simulink model "TosAeb"  (R2021a)
settings: Solver=FixedStepDiscrete  StartTime=0.0  StopTime=inf  FixedStep=0.01  RelTol=1e-3
undefined symbols (not defined in the file or in its folder): ACCModSts, AccSts1, AEB_ObjectMovingOrientation, AEB_ObjectMovingState, CN_BTCActive, CN_CurrentGearR, CN_CurrentGearR_DHT, CN_PTCActive, CN_VDCActive, CN_VSC_EspOff, CN_VSC_FCW_AEB_Error, CN_VSC_FCW_AEB_NoDispay, CN_VSC_FCW_AEB_PerfmDgdtn, CN_VSC_FCW_AEB_TempUnAvlbl, CN_VSC_VehStandstill, CNaccelAvoidMax, CNaccelAvoidMin, CNbrakePedalPress, CNcomfortStrLeftAccelMax, CNcomfortStrLeftAccelMin, CNcomfortStrRightAccelMax, CNcomfortStrRightAccelMin, CNDrvDoorOpen, CNDrvModeEco, CNDrvModeNorm, CNDrvModeOther, CNDrvModeSport, CNDrvSBRreminder, CNNull, CNobjInvalidID, CNPassDoorOpen, CNPassSBRreminder, CNPEBSAccelNegInf, CNPEBSAccelNull, CNPEBSTimeInf, CNPEBSTimeNull, CNSTOPMAX, CNSTOPNULL, CNsubjWidth, CNSwtOFF, CNSwtON, CNTTCMAX, CNTTCNULL, CNVehConfigAEBCycNone, CNVehConfigAEBNone, CNVehConfigAEBPedNone, CNVehConfigFCWNone, CNVehConfigJANone, CollisionSeverity, FALSE, FCWSnvty, JACycObjCalcInfoDefaultParams, JAPedObjCalcInfoDefaultParams, JAVehObjCalcInfoDefaultParams, latCycObjCalcInfoDefaultParams, latPedObjCalcInfoDefaultParams, longObjCalcInfoDefaultParams, ModSts, movingStatus, ObjectType ... (+1112)
libraries: simulink x3031, AEBSFunctionLib x50, Ta_Library x33, AEBSStateMachineLib x23, Mylibrary x12, simulink_extras x7, ObjSelect_lib x3, dspstat3 x2
(part 7 of 102)

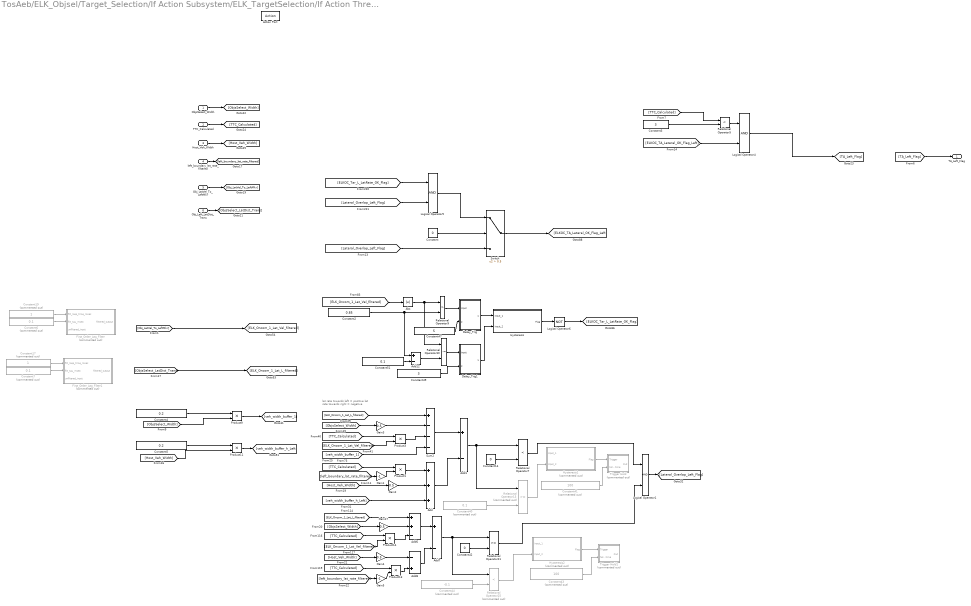
== system TosAeb/ELK_Objsel/Target_Selection/If Action Subsystem/ELK_TargetSelection/If Action Threat_Assessment_Left1 ==
  note: lat rate towards left = positive
      | lat rate towards right = negative
  ObjsSelect_Width : Inport
  TTC_Calculated : Inport  Port=2
  Host_Veh_Width : Inport  Port=3
  left_boundary_lat_rate_filtered : Inport  Port=4
  Obj_LatVel_To_LeftMkr : Inport  Port=5
  Obj_Left_LatDist_Trans : Inport  Port=6
  Action Port : ActionPort  ActionPortLabel=if(u1 ~= 0)
  Abs : Abs  Description=_GWMH9P4.ELK.000_40_
  Add : Sum  Description=_GWMH9P5.ELK.000_14_  Inputs=+++
  Add1 : Sum  Description=_GWMH9P5.ELK.000_15_  Inputs=+-
  Add12 : Sum  Description=_GWMH9P5.ELK.000_18_  Inputs=+-
  Add6 : Sum  Description=_GWMH9P5.ELK.000_22_  Inputs=++-
  Add7 : Sum  Description=_GWMH9P5.ELK.000_23_  Inputs=+-
  Add8 : Sum  Description=_GWMH9P5.ELK.000_24_  Inputs=-+
  Constant : Constant  Value=0
  Constant14 : Constant  Description=_GWMH9P4.ELK.000_42_  Value=0
  Constant17 : Constant  Description=_GWMH9P5.ELK.000_26_  Commented=on  OutDataTypeStr=single
  Constant19 : Constant  Description=_GWMH9P5.ELK.000_27_  Commented=on  OutDataTypeStr=single
  Constant2 : Constant  Description=_GWMH9P5.ELK.000_52_  Value=0.85
  Constant3 : Constant  Description=_GWMH9P5.ELK.000_38_  Value=3
  Constant4 : Constant  Description=_GWMH9P5.ELK.000_45_  Value=0.2
  Constant40 : Constant  Description=_GWMH9P5.ELK.000_41_  Commented=on  Value=0.1  mask(TL_Constant){data={'date',738384.81607121532}}
      settings: CopyFcn=if exist('tl_reset_reviewflag','file')==2,tl_reset_reviewflag(gcbh);end  DeleteFcn=if exist('delete_tlblock','file')==2,delete_tlblock(gcbh);end  NameChangeFcn=if exist('rename_tlblock','file')==2,rename_tlblock(gcbh);end  OpenFcn=if exist('tl_open_blockdlg','file')==2,tl_open_blockdlg(gcbh);else,open_system(gcbh,'parameter');end
      mask Help: eval(['if exist(''tl_online_help'',''file'') == 2, tl_online_help(''Topic'',''TL_Constant''),else, web([''jar:file:///'',matlabroot,''/help/toolbox/simulink/help.jar!/slref/' lower(get_param(gcbh,'Blocktype')) '.html''], ''-helpbrowser''),end'])
      mask Display: plot([-0.1, 1.05, NaN, -0.1, 1.05],[.01, .01, NaN, .99, .99]);
  Constant41 : Constant  Description=_GWMH9P5.ELK.000_42_  Commented=on  Value=100  mask(TL_Constant){data={'date',738384.81607467588}}
      settings: CopyFcn=if exist('tl_reset_reviewflag','file')==2,tl_reset_reviewflag(gcbh);end  DeleteFcn=if exist('delete_tlblock','file')==2,delete_tlblock(gcbh);end  NameChangeFcn=if exist('rename_tlblock','file')==2,rename_tlblock(gcbh);end  OpenFcn=if exist('tl_open_blockdlg','file')==2,tl_open_blockdlg(gcbh);else,open_system(gcbh,'parameter');end
      mask Help: eval(['if exist(''tl_online_help'',''file'') == 2, tl_online_help(''Topic'',''TL_Constant''),else, web([''jar:file:///'',matlabroot,''/help/toolbox/simulink/help.jar!/slref/' lower(get_param(gcbh,'Blocktype')) '.html''], ''-helpbrowser''),end'])
      mask Display: plot([-0.1, 1.05, NaN, -0.1, 1.05],[.01, .01, NaN, .99, .99]);
  Constant42 : Constant  Description=_GWMH9P5.ELK.000_43_  Value=0
  Constant43 : Constant  Description=_GWMH9P5.ELK.000_44_  Commented=on  Value=100  mask(TL_Constant){data={'date',738384.81608211808}}
      settings: CopyFcn=if exist('tl_reset_reviewflag','file')==2,tl_reset_reviewflag(gcbh);end  DeleteFcn=if exist('delete_tlblock','file')==2,delete_tlblock(gcbh);end  NameChangeFcn=if exist('rename_tlblock','file')==2,rename_tlblock(gcbh);end  OpenFcn=if exist('tl_open_blockdlg','file')==2,tl_open_blockdlg(gcbh);else,open_system(gcbh,'parameter');end
      mask Help: eval(['if exist(''tl_online_help'',''file'') == 2, tl_online_help(''Topic'',''TL_Constant''),else, web([''jar:file:///'',matlabroot,''/help/toolbox/simulink/help.jar!/slref/' lower(get_param(gcbh,'Blocktype')) '.html''], ''-helpbrowser''),end'])
      mask Display: plot([-0.1, 1.05, NaN, -0.1, 1.05],[.01, .01, NaN, .99, .99]);
  Constant44 : Constant  Description=_GWMH9P5.ELK.000_45_  Commented=on  Value=-0.1  mask(TL_Constant){data={'date',738384.81608572917}}
      settings: CopyFcn=if exist('tl_reset_reviewflag','file')==2,tl_reset_reviewflag(gcbh);end  DeleteFcn=if exist('delete_tlblock','file')==2,delete_tlblock(gcbh);end  NameChangeFcn=if exist('rename_tlblock','file')==2,rename_tlblock(gcbh);end  OpenFcn=if exist('tl_open_blockdlg','file')==2,tl_open_blockdlg(gcbh);else,open_system(gcbh,'parameter');end
      mask Help: eval(['if exist(''tl_online_help'',''file'') == 2, tl_online_help(''Topic'',''TL_Constant''),else, web([''jar:file:///'',matlabroot,''/help/toolbox/simulink/help.jar!/slref/' lower(get_param(gcbh,'Blocktype')) '.html''], ''-helpbrowser''),end'])
      mask Display: plot([-0.1, 1.05, NaN, -0.1, 1.05],[.01, .01, NaN, .99, .99]);
  Constant47 : Constant  Description=_GWMH9P5.ELK.000_48_  Value=5
  Constant49 : Constant  Description=_GWMH9P5.ELK.000_50_  Value=3
  Constant5 : Constant  Description=_GWMH9P5.ELK.000_45_  Value=0.2
  Constant51 : Constant  Description=_GWMH9P5.ELK.000_52_  Value=0.1
  Constant6 : Constant  Description=_GWMH9P5.ELK.000_27_  Commented=on  Value=0.1  OutDataTypeStr=single
  Constant7 : Constant  Description=_GWMH9P5.ELK.000_27_  Commented=on  Value=0.1  OutDataTypeStr=single
  Delay_Trig : Subsystem
  Delay_Trig1 : Subsystem
  First_Order_Lag_Filter : Subsystem  Commented=on
  First_Order_Lag_Filter1 : Subsystem  Commented=on
  Gain1 : Gain  Description=_GWMH9P4.ELK.000_64_  Gain=-1
  Gain17 : Gain  Description=_GWMH9P5.ELK.000_80_  Gain=0.5
  Gain2 : Gain  Description=_GWMH9P4.ELK.000_64_  Gain=0.5
  Gain3 : Gain  Description=_GWMH9P4.ELK.000_64_  Gain=0.5
  Gain4 : Gain  Description=_GWMH9P4.ELK.000_64_  Gain=0.5
  Gain5 : Gain  Description=_GWMH9P4.ELK.000_64_  Gain=-1
  Hysteresis : Stateflow chart  TreatAsAtomicUnit=on
  Hysteresis1 : Stateflow chart  Commented=on  TreatAsAtomicUnit=on
  Hysteresis2 : Stateflow chart  Commented=on  TreatAsAtomicUnit=on
  Logical Operator1 : Logic  Description=_GWMH9P5.ELK.000_86_  AllPortsSameDT=off  OutDataTypeStr=boolean
  Logical Operator4 : Logic  Description=_GWMH9P4.ELK.000_68_  AllPortsSameDT=off  OutDataTypeStr=boolean
  Logical Operator5 : Logic  Description=_GWMH9P4.ELK.000_68_  AllPortsSameDT=off  OutDataTypeStr=boolean
  Logical Operator6 : Logic  Description=_GWMH9P5.ELK.000_88_  Operator=NOT  Inputs=1  AllPortsSameDT=off  OutDataTypeStr=boolean
  Product1 : Product  Description=_GWMH9P4.ELK.000_71_  RndMeth=Zero
  Product11 : Product  Description=_GWMH9P5.ELK.000_91_
  Product13 : Product  Description=_GWMH9P5.ELK.000_93_  RndMeth=Zero
  Product14 : Product  Description=_GWMH9P5.ELK.000_94_  RndMeth=Zero
  Product3 : Product  Description=_GWMH9P4.ELK.000_73_  RndMeth=Zero
  Product9 : Product  Description=_GWMH9P5.ELK.000_102_
  Relational Operator13 : RelationalOperator  Description=_GWMH9P5.ELK.000_106_  Commented=on  OutDataTypeStr=boolean  RndMeth=Nearest  mask(TL_RelationalOperator){data={'date',738384.81682939816}}
      settings: DeleteFcn=if exist('delete_tlblock','file')==2,delete_tlblock(gcbh);end  NameChangeFcn=if exist('rename_tlblock','file')==2,rename_tlblock(gcbh);end  OpenFcn=if exist('tl_open_blockdlg','file')==2,tl_open_blockdlg(gcbh);else,open_system(gcbh,'parameter');end
      mask Help: eval(['if exist(''tl_online_help'',''file'') == 2, tl_online_help(''Topic'',''TL_RelationalOperator''),else, web([''jar:file:///'',matlabroot,''/help/toolbox/simulink/help.jar!/slref/' lower(get_param(gcbh,'Blocktype')) '.html''], ''-helpbrowser''),end'])
      mask Display: plot([-0.1, 1.05, NaN, -0.1, 1.05],[.01, .01, NaN, .99, .99]);
  Relational Operator21 : RelationalOperator  Description=_GWMH9P5.ELK.000_108_  OutDataTypeStr=boolean  RndMeth=Nearest
  Relational Operator25 : RelationalOperator  Description=_GWMH9P5.ELK.000_109_  Commented=on  Operator=<  OutDataTypeStr=boolean  RndMeth=Nearest  mask(TL_RelationalOperator){data={'date',738384.8168361343}}
      settings: DeleteFcn=if exist('delete_tlblock','file')==2,delete_tlblock(gcbh);end  NameChangeFcn=if exist('rename_tlblock','file')==2,rename_tlblock(gcbh);end  OpenFcn=if exist('tl_open_blockdlg','file')==2,tl_open_blockdlg(gcbh);else,open_system(gcbh,'parameter');end
      mask Help: eval(['if exist(''tl_online_help'',''file'') == 2, tl_online_help(''Topic'',''TL_RelationalOperator''),else, web([''jar:file:///'',matlabroot,''/help/toolbox/simulink/help.jar!/slref/' lower(get_param(gcbh,'Blocktype')) '.html''], ''-helpbrowser''),end'])
      mask Display: plot([-0.1, 1.05, NaN, -0.1, 1.05],[.01, .01, NaN, .99, .99]);
  Relational Operator30 : RelationalOperator  Description=_GWMH9P5.ELK.000_112_  Operator=<=  OutDataTypeStr=boolean  RndMeth=Nearest
  Relational Operator5 : RelationalOperator  Description=_GWMH9P3.ELK.000_91_  Operator=<  OutDataTypeStr=boolean  RndMeth=Nearest
  Relational Operator7 : RelationalOperator  Description=_GWMH9P4.ELK.000_81_  Operator=<  OutDataTypeStr=boolean  RndMeth=Nearest
  Relational Operator9 : RelationalOperator  Description=_GWMH9P5.ELK.000_114_  Operator=>  OutDataTypeStr=boolean  RndMeth=Nearest
  Sum2 : Sum  Description=_GWMH9P4.ELK.000_83_  Inputs=+---
  Switch : Switch  Criteria=u2 > Threshold  Threshold=0.5
  Trigger Hold : Subsystem  Commented=on
  Trigger Hold1 : Subsystem  Commented=on
  TA_Left_Flag : Outport
  wires:
  Logical Operator6/1 -> [ELKOC_Tar_L_LatRate_OK_Flag]
  [Lateral_Overlap_Left_Flag] -> Switch/3
  Constant/1 -> Switch/2
  Switch/1 -> [ELKOC_TA_Lateral_OK_Flag_Left]
  Logical Operator5/1 -> Switch/1
  Gain5/1 -> Product13/2
  [Host_Veh_Width] -> Gain4/1
  Gain2/1 -> Add/2
  Gain1/1 -> Product1/2
  Obj_Left_LatDist_Trans/1 -> [ObjsSelect_LatDist_Trans]
  Delay_Trig/1(Output) -> Hysteresis/1(Input_1)
  Constant5/1 -> Product11/1
  [Host_Veh_Width] -> Product11/2
  Product11/1 -> [veh_width_buffer_h_Left]
  Product9/1 -> [veh_width_buffer_1]
  Constant4/1 -> Product9/1
  [ObjsSelect_Width] -> Product9/2
  [ObjsSelect_LatDist_Trans] -> [ELK_Oncom_1_Lat_L_filtered]
  [TTC_Calculated] -> Product1/1
  [ObjsSelect_Width] -> Gain3/1
  [left_boundary_lat_rate_filtered] -> Gain1/1
  Constant14/1 -> Relational Operator7/2
  [TTC_Calculated] -> Product3/1
  [ELK_Oncom_1_Lat_Vel_filtered] -> Product3/2
  [ELK_Oncom_1_Lat_L_filtered] -> Sum2/1
  Gain3/1 -> Sum2/2
  Product3/1 -> Sum2/3
  [veh_width_buffer_1] -> Sum2/4
  Product1/1 -> Add/1
  [Host_Veh_Width] -> Gain2/1
  [veh_width_buffer_h_Left] -> Add/3
  Sum2/1 -> Add1/1
  Add/1 -> Add1/2
  Add1/1 -> Relational Operator13/1, Relational Operator7/1
  Logical Operator1/1 -> [Lateral_Overlap_Left_Flag]
  Hysteresis1/1(Flag) -> Trigger Hold/1(Trigger)
  Relational Operator7/1 -> Logical Operator1/1
  Constant40/1 -> Relational Operator13/2
  Relational Operator13/1 -> Hysteresis1/2(Input_2)
  Constant41/1 -> Trigger Hold/2(hold_time)
  [ObjsSelect_Width] -> Gain17/1
  Gain17/1 -> Add6/2
  [TTC_Calculated] -> Product14/1
  [ELK_Oncom_1_Lat_Vel_filtered] -> Product14/2
  Product14/1 -> Add6/3
  Add6/1 -> Add7/1
  [TTC_Calculated] -> Product13/1
  [left_boundary_lat_rate_filtered] -> Gain5/1
  Add7/1 -> Relational Operator25/1, Relational Operator21/1
  Constant42/1 -> Relational Operator21/2
  [ELK_Oncom_1_Lat_L_filtered] -> Add6/1
  Product13/1 -> Add8/2
  Gain4/1 -> Add8/1
  Add8/1 -> Add7/2
  Constant44/1 -> Relational Operator25/2
  Relational Operator21/1 -> Logical Operator1/2
  Relational Operator25/1 -> Hysteresis2/2(Input_2)
  Hysteresis2/1(Flag) -> Trigger Hold1/1(Trigger)
  Constant43/1 -> Trigger Hold1/2(hold_time)
  [Obj_LatVel_To_LeftMkr] -> [ELK_Oncom_1_Lat_Vel_filtered]
  [ELK_Oncom_1_Lat_Vel_filtered] -> Abs/1
  Constant2/1 -> Add12/1, Relational Operator9/2
  Constant47/1 -> Delay_Trig/2(Delay_Cnt)
  Relational Operator30/1 -> Delay_Trig1/1(Input)
  Delay_Trig1/1(Output) -> Hysteresis/2(Input_2)
  Constant49/1 -> Delay_Trig1/2(Delay_Cnt)
  Abs/1 -> Relational Operator30/1, Relational Operator9/1
  Add12/1 -> Relational Operator30/2
  Constant51/1 -> Add12/2
  Relational Operator9/1 -> Delay_Trig/1(Input)
  Hysteresis/1(Flag) -> Logical Operator6/1
  Obj_LatVel_To_LeftMkr/1 -> [Obj_LatVel_To_LeftMkr]
  left_boundary_lat_rate_filtered/1 -> [left_boundary_lat_rate_filtered]
  Host_Veh_Width/1 -> [Host_Veh_Width]
  TTC_Calculated/1 -> [TTC_Calculated]
  ObjsSelect_Width/1 -> [ObjsSelect_Width]
  [TA_Left_Flag] -> TA_Left_Flag/1
  [Lateral_Overlap_Left_Flag] -> Logical Operator5/2
  [ELKOC_Tar_L_LatRate_OK_Flag] -> Logical Operator5/1
  Relational Operator5/1 -> Logical Operator4/1
  [TTC_Calculated] -> Relational Operator5/1
  [ELKOC_TA_Lateral_OK_Flag_Left] -> Logical Operator4/2
  Constant3/1 -> Relational Operator5/2
  Logical Operator4/1 -> [TA_Left_Flag]
  Constant6/1 -> First_Order_Lag_Filter/2(filt_tau_msec)
  Constant19/1 -> First_Order_Lag_Filter/1(filt_loop_time_msec)
  Constant7/1 -> First_Order_Lag_Filter1/2(filt_tau_msec)
  Constant17/1 -> First_Order_Lag_Filter1/1(filt_loop_time_msec)

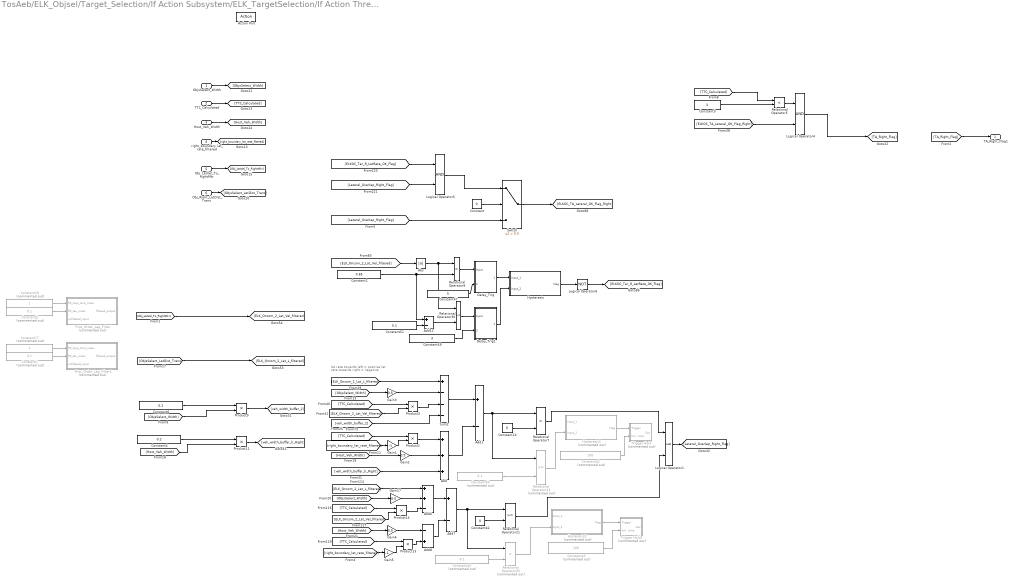
== system TosAeb/ELK_Objsel/Target_Selection/If Action Subsystem/ELK_TargetSelection/If Action Threat_Assessment_Right ==
  note: lat rate towards left = positive
      | lat rate towards right = negative
  ObjsSelect_Width : Inport
  TTC_Calculated : Inport  Port=2
  Host_Veh_Width : Inport  Port=3
  right_boundary_lat_rate_filtered : Inport  Port=4
  Obj_LatVel_To_RightMkr : Inport  Port=5
  Obj_Right_LatDist_Trans : Inport  Port=6
  Action Port : ActionPort  ActionPortLabel=if(u1 ~= 0)
  Abs : Abs  Description=_GWMH9P4.ELK.000_40_
  Add : Sum  Description=_GWMH9P5.ELK.000_14_  Inputs=+++
  Add1 : Sum  Description=_GWMH9P5.ELK.000_15_  Inputs=+-
  Add12 : Sum  Description=_GWMH9P5.ELK.000_18_  Inputs=+-
  Add6 : Sum  Description=_GWMH9P5.ELK.000_22_  Inputs=++-
  Add7 : Sum  Description=_GWMH9P5.ELK.000_23_  Inputs=+-
  Add8 : Sum  Description=_GWMH9P5.ELK.000_24_  Inputs=-+
  Constant : Constant  Value=0
  Constant14 : Constant  Description=_GWMH9P4.ELK.000_42_  Value=0
  Constant17 : Constant  Description=_GWMH9P5.ELK.000_26_  Commented=on
  Constant19 : Constant  Description=_GWMH9P5.ELK.000_27_  Commented=on
  Constant2 : Constant  Description=_GWMH9P5.ELK.000_52_  Value=0.85
  Constant3 : Constant  Description=_GWMH9P5.ELK.000_38_  Value=3
  Constant4 : Constant  Description=_GWMH9P5.ELK.000_52_  Value=0.2
  Constant40 : Constant  Description=_GWMH9P5.ELK.000_41_  Commented=on  Value=0.1  mask(TL_Constant){data={'date',738384.85978439811}}
      settings: CopyFcn=if exist('tl_reset_reviewflag','file')==2,tl_reset_reviewflag(gcbh);end  DeleteFcn=if exist('delete_tlblock','file')==2,delete_tlblock(gcbh);end  NameChangeFcn=if exist('rename_tlblock','file')==2,rename_tlblock(gcbh);end  OpenFcn=if exist('tl_open_blockdlg','file')==2,tl_open_blockdlg(gcbh);else,open_system(gcbh,'parameter');end
      mask Help: eval(['if exist(''tl_online_help'',''file'') == 2, tl_online_help(''Topic'',''TL_Constant''),else, web([''jar:file:///'',matlabroot,''/help/toolbox/simulink/help.jar!/slref/' lower(get_param(gcbh,'Blocktype')) '.html''], ''-helpbrowser''),end'])
      mask Display: plot([-0.1, 1.05, NaN, -0.1, 1.05],[.01, .01, NaN, .99, .99]);
  Constant41 : Constant  Description=_GWMH9P5.ELK.000_42_  Commented=on  Value=100  mask(TL_Constant){data={'date',738384.85978776624}}
      settings: CopyFcn=if exist('tl_reset_reviewflag','file')==2,tl_reset_reviewflag(gcbh);end  DeleteFcn=if exist('delete_tlblock','file')==2,delete_tlblock(gcbh);end  NameChangeFcn=if exist('rename_tlblock','file')==2,rename_tlblock(gcbh);end  OpenFcn=if exist('tl_open_blockdlg','file')==2,tl_open_blockdlg(gcbh);else,open_system(gcbh,'parameter');end
      mask Help: eval(['if exist(''tl_online_help'',''file'') == 2, tl_online_help(''Topic'',''TL_Constant''),else, web([''jar:file:///'',matlabroot,''/help/toolbox/simulink/help.jar!/slref/' lower(get_param(gcbh,'Blocktype')) '.html''], ''-helpbrowser''),end'])
      mask Display: plot([-0.1, 1.05, NaN, -0.1, 1.05],[.01, .01, NaN, .99, .99]);
  Constant42 : Constant  Description=_GWMH9P5.ELK.000_43_  Value=0
  Constant43 : Constant  Description=_GWMH9P5.ELK.000_44_  Commented=on  Value=100  mask(TL_Constant){data={'date',738384.85979500005}}
      settings: CopyFcn=if exist('tl_reset_reviewflag','file')==2,tl_reset_reviewflag(gcbh);end  DeleteFcn=if exist('delete_tlblock','file')==2,delete_tlblock(gcbh);end  NameChangeFcn=if exist('rename_tlblock','file')==2,rename_tlblock(gcbh);end  OpenFcn=if exist('tl_open_blockdlg','file')==2,tl_open_blockdlg(gcbh);else,open_system(gcbh,'parameter');end
      mask Help: eval(['if exist(''tl_online_help'',''file'') == 2, tl_online_help(''Topic'',''TL_Constant''),else, web([''jar:file:///'',matlabroot,''/help/toolbox/simulink/help.jar!/slref/' lower(get_param(gcbh,'Blocktype')) '.html''], ''-helpbrowser''),end'])
      mask Display: plot([-0.1, 1.05, NaN, -0.1, 1.05],[.01, .01, NaN, .99, .99]);
  Constant44 : Constant  Description=_GWMH9P5.ELK.000_45_  Commented=on  Value=-0.1  mask(TL_Constant){data={'date',738384.85979850695}}
      settings: CopyFcn=if exist('tl_reset_reviewflag','file')==2,tl_reset_reviewflag(gcbh);end  DeleteFcn=if exist('delete_tlblock','file')==2,delete_tlblock(gcbh);end  NameChangeFcn=if exist('rename_tlblock','file')==2,rename_tlblock(gcbh);end  OpenFcn=if exist('tl_open_blockdlg','file')==2,tl_open_blockdlg(gcbh);else,open_system(gcbh,'parameter');end
      mask Help: eval(['if exist(''tl_online_help'',''file'') == 2, tl_online_help(''Topic'',''TL_Constant''),else, web([''jar:file:///'',matlabroot,''/help/toolbox/simulink/help.jar!/slref/' lower(get_param(gcbh,'Blocktype')) '.html''], ''-helpbrowser''),end'])
      mask Display: plot([-0.1, 1.05, NaN, -0.1, 1.05],[.01, .01, NaN, .99, .99]);
  Constant47 : Constant  Description=_GWMH9P5.ELK.000_48_  Value=5
  Constant49 : Constant  Description=_GWMH9P5.ELK.000_50_  Value=3
  Constant5 : Constant  Description=_GWMH9P5.ELK.000_27_  Commented=on  Value=0.1
  Constant51 : Constant  Description=_GWMH9P5.ELK.000_52_  Value=0.1
  Constant6 : Constant  Description=_GWMH9P5.ELK.000_52_  Value=0.2
  Constant7 : Constant  Description=_GWMH9P5.ELK.000_27_  Commented=on  Value=0.1
  Delay_Trig : Subsystem
  Delay_Trig1 : Subsystem
  First_Order_Lag_Filter : Subsystem  Commented=on
  First_Order_Lag_Filter1 : Subsystem  Commented=on
  Gain1 : Gain  Description=_GWMH9P4.ELK.000_64_  Gain=-1
  Gain17 : Gain  Description=_GWMH9P5.ELK.000_80_  Gain=0.5
  Gain2 : Gain  Description=_GWMH9P4.ELK.000_64_  Gain=0.5
  Gain3 : Gain  Description=_GWMH9P4.ELK.000_64_  Gain=0.5
  Gain4 : Gain  Description=_GWMH9P4.ELK.000_64_  Gain=0.5
  Gain5 : Gain  Description=_GWMH9P4.ELK.000_64_  Gain=-1
  Hysteresis : Stateflow chart  TreatAsAtomicUnit=on
  Hysteresis1 : Stateflow chart  Commented=on  TreatAsAtomicUnit=on
  Hysteresis2 : Stateflow chart  Commented=on  TreatAsAtomicUnit=on
  Logical Operator1 : Logic  Description=_GWMH9P5.ELK.000_86_  AllPortsSameDT=off  OutDataTypeStr=boolean
  Logical Operator4 : Logic  Description=_GWMH9P4.ELK.000_68_  AllPortsSameDT=off  OutDataTypeStr=boolean
  Logical Operator5 : Logic  Description=_GWMH9P4.ELK.000_68_  AllPortsSameDT=off  OutDataTypeStr=boolean
  Logical Operator6 : Logic  Description=_GWMH9P5.ELK.000_88_  Operator=NOT  Inputs=1  AllPortsSameDT=off  OutDataTypeStr=boolean
  Product1 : Product  Description=_GWMH9P4.ELK.000_71_  RndMeth=Zero
  Product11 : Product  Description=_GWMH9P5.ELK.000_91_
  Product13 : Product  Description=_GWMH9P5.ELK.000_93_  RndMeth=Zero
  Product14 : Product  Description=_GWMH9P5.ELK.000_94_  RndMeth=Zero
  Product3 : Product  Description=_GWMH9P4.ELK.000_73_  RndMeth=Zero
  Product9 : Product  Description=_GWMH9P5.ELK.000_102_
  Relational Operator13 : RelationalOperator  Description=_GWMH9P5.ELK.000_106_  Commented=on  OutDataTypeStr=boolean  RndMeth=Nearest  mask(TL_RelationalOperator){data={'date',738384.85993935179}}
      settings: DeleteFcn=if exist('delete_tlblock','file')==2,delete_tlblock(gcbh);end  NameChangeFcn=if exist('rename_tlblock','file')==2,rename_tlblock(gcbh);end  OpenFcn=if exist('tl_open_blockdlg','file')==2,tl_open_blockdlg(gcbh);else,open_system(gcbh,'parameter');end
      mask Help: eval(['if exist(''tl_online_help'',''file'') == 2, tl_online_help(''Topic'',''TL_RelationalOperator''),else, web([''jar:file:///'',matlabroot,''/help/toolbox/simulink/help.jar!/slref/' lower(get_param(gcbh,'Blocktype')) '.html''], ''-helpbrowser''),end'])
      mask Display: plot([-0.1, 1.05, NaN, -0.1, 1.05],[.01, .01, NaN, .99, .99]);
  Relational Operator21 : RelationalOperator  Description=_GWMH9P5.ELK.000_108_  OutDataTypeStr=boolean  RndMeth=Nearest
  Relational Operator25 : RelationalOperator  Description=_GWMH9P5.ELK.000_109_  Commented=on  Operator=<  OutDataTypeStr=boolean  RndMeth=Nearest  mask(TL_RelationalOperator){data={'date',738384.85994590283}}
      settings: DeleteFcn=if exist('delete_tlblock','file')==2,delete_tlblock(gcbh);end  NameChangeFcn=if exist('rename_tlblock','file')==2,rename_tlblock(gcbh);end  OpenFcn=if exist('tl_open_blockdlg','file')==2,tl_open_blockdlg(gcbh);else,open_system(gcbh,'parameter');end
      mask Help: eval(['if exist(''tl_online_help'',''file'') == 2, tl_online_help(''Topic'',''TL_RelationalOperator''),else, web([''jar:file:///'',matlabroot,''/help/toolbox/simulink/help.jar!/slref/' lower(get_param(gcbh,'Blocktype')) '.html''], ''-helpbrowser''),end'])
      mask Display: plot([-0.1, 1.05, NaN, -0.1, 1.05],[.01, .01, NaN, .99, .99]);
  Relational Operator30 : RelationalOperator  Description=_GWMH9P5.ELK.000_112_  Operator=<=  OutDataTypeStr=boolean  RndMeth=Nearest
  Relational Operator5 : RelationalOperator  Description=_GWMH9P3.ELK.000_91_  Operator=<  OutDataTypeStr=boolean  RndMeth=Nearest
  Relational Operator7 : RelationalOperator  Description=_GWMH9P4.ELK.000_81_  Operator=<  OutDataTypeStr=boolean  RndMeth=Nearest
  Relational Operator9 : RelationalOperator  Description=_GWMH9P5.ELK.000_114_  Operator=>  OutDataTypeStr=boolean  RndMeth=Nearest
  Sum2 : Sum  Description=_GWMH9P4.ELK.000_83_  Inputs=+---
  Switch : Switch  Criteria=u2 > Threshold  Threshold=0.5
  Trigger Hold : Subsystem  Commented=on
  Trigger Hold1 : Subsystem  Commented=on
  TA_Right_Flag1 : Outport
  wires:
  Constant3/1 -> Relational Operator5/2
  [ELKOC_TA_Lateral_OK_Flag_Right] -> Logical Operator4/2
  [TTC_Calculated] -> Relational Operator5/1
  Logical Operator4/1 -> [TA_Right_Flag]
  Relational Operator5/1 -> Logical Operator4/1
  [ELKOC_Tar_R_LatRate_OK_Flag] -> Logical Operator5/1
  Logical Operator5/1 -> Switch/1
  [Lateral_Overlap_Right_Flag] -> Logical Operator5/2
  [TA_Right_Flag] -> TA_Right_Flag1/1
  ObjsSelect_Width/1 -> [ObjsSelect_Width]
  TTC_Calculated/1 -> [TTC_Calculated]
  Host_Veh_Width/1 -> [Host_Veh_Width]
  right_boundary_lat_rate_filtered/1 -> [right_boundary_lat_rate_filtered]
  Obj_LatVel_To_RightMkr/1 -> [Obj_LatVel_To_RightMkr]
  Hysteresis/1(Flag) -> Logical Operator6/1
  Relational Operator9/1 -> Delay_Trig/1(Input)
  Constant51/1 -> Add12/2
  Add12/1 -> Relational Operator30/2
  Abs/1 -> Relational Operator9/1, Relational Operator30/1
  Constant49/1 -> Delay_Trig1/2(Delay_Cnt)
  Delay_Trig1/1(Output) -> Hysteresis/2(Input_2)
  Relational Operator30/1 -> Delay_Trig1/1(Input)
  Constant47/1 -> Delay_Trig/2(Delay_Cnt)
  Constant2/1 -> Relational Operator9/2, Add12/1
  Logical Operator6/1 -> [ELKOC_Tar_R_LatRate_OK_Flag]
  [ELK_Oncom_2_Lat_Vel_filtered] -> Abs/1
  [Obj_LatVel_To_RightMkr] -> [ELK_Oncom_2_Lat_Vel_filtered]
  Constant43/1 -> Trigger Hold1/2(hold_time)
  Hysteresis2/1(Flag) -> Trigger Hold1/1(Trigger)
  Relational Operator25/1 -> Hysteresis2/2(Input_2)
  Relational Operator21/1 -> Logical Operator1/2
  Constant44/1 -> Relational Operator25/2
  Add8/1 -> Add7/2
  Gain4/1 -> Add8/1
  Product13/1 -> Add8/2
  [ELK_Oncom_2_Lat_L_filtered] -> Add6/1
  Constant42/1 -> Relational Operator21/2
  Add7/1 -> Relational Operator21/1, Relational Operator25/1
  [right_boundary_lat_rate_filtered] -> Gain5/1
  [TTC_Calculated] -> Product13/1
  Add6/1 -> Add7/1
  Product14/1 -> Add6/3
  [ELK_Oncom_2_Lat_Vel_filtered] -> Product14/2
  [TTC_Calculated] -> Product14/1
  Gain17/1 -> Add6/2
  [ObjsSelect_Width] -> Gain17/1
  Constant41/1 -> Trigger Hold/2(hold_time)
  Relational Operator13/1 -> Hysteresis1/2(Input_2)
  Constant40/1 -> Relational Operator13/2
  Relational Operator7/1 -> Logical Operator1/1
  Hysteresis1/1(Flag) -> Trigger Hold/1(Trigger)
  Logical Operator1/1 -> [Lateral_Overlap_Right_Flag]
  Add1/1 -> Relational Operator7/1, Relational Operator13/1
  Add/1 -> Add1/2
  Sum2/1 -> Add1/1
  [veh_width_buffer_h_Right] -> Add/3
  [Host_Veh_Width] -> Gain2/1
  Product1/1 -> Add/1
  [veh_width_buffer_2] -> Sum2/4
  Product3/1 -> Sum2/3
  Gain3/1 -> Sum2/2
  [ELK_Oncom_2_Lat_Vel_filtered] -> Product3/2
  [TTC_Calculated] -> Product3/1
  Constant14/1 -> Relational Operator7/2
  [right_boundary_lat_rate_filtered] -> Gain1/1
  [ObjsSelect_Width] -> Gain3/1
  [TTC_Calculated] -> Product1/1
  [ObjsSelect_LatDist_Trans] -> [ELK_Oncom_2_Lat_L_filtered]
  [ObjsSelect_Width] -> Product9/2
  Constant6/1 -> Product9/1
  Product9/1 -> [veh_width_buffer_2]
  Product11/1 -> [veh_width_buffer_h_Right]
  [Host_Veh_Width] -> Product11/2
  Constant4/1 -> Product11/1
  Delay_Trig/1(Output) -> Hysteresis/1(Input_1)
  Obj_Right_LatDist_Trans/1 -> [ObjsSelect_LatDist_Trans]
  Gain1/1 -> Product1/2
  Gain2/1 -> Add/2
  [Host_Veh_Width] -> Gain4/1
  Gain5/1 -> Product13/2
  Switch/1 -> [ELKOC_TA_Lateral_OK_Flag_Right]
  Constant/1 -> Switch/2
  [Lateral_Overlap_Right_Flag] -> Switch/3
  Constant19/1 -> First_Order_Lag_Filter/1(filt_loop_time_msec)
  Constant5/1 -> First_Order_Lag_Filter/2(filt_tau_msec)
  Constant17/1 -> First_Order_Lag_Filter1/1(filt_loop_time_msec)
  Constant7/1 -> First_Order_Lag_Filter1/2(filt_tau_msec)
  [ELK_Oncom_2_Lat_L_filtered] -> Sum2/1

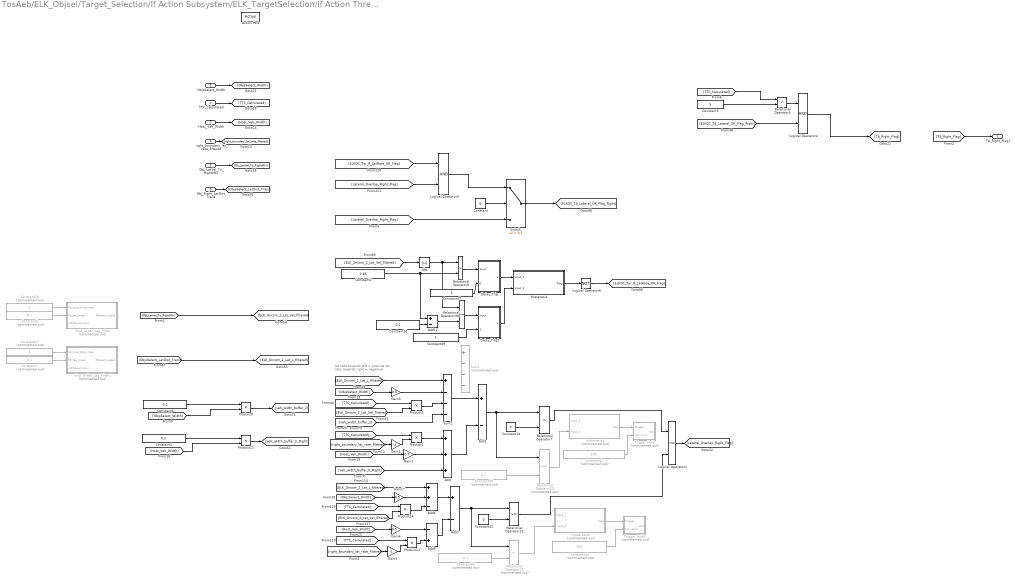
== system TosAeb/ELK_Objsel/Target_Selection/If Action Subsystem/ELK_TargetSelection/If Action Threat_Assessment_Right1 ==
  note: lat rate towards left = positive
      | lat rate towards right = negative
  ObjsSelect_Width : Inport
  TTC_Calculated : Inport  Port=2
  Host_Veh_Width : Inport  Port=3
  right_boundary_lat_rate_filtered : Inport  Port=4
  Obj_LatVel_To_RightMkr : Inport  Port=5
  Obj_Right_LatDist_Trans : Inport  Port=6
  Action Port : ActionPort  ActionPortLabel=if(u1 ~= 0)
  Abs : Abs  Description=_GWMH9P4.ELK.000_40_
  Add : Sum  Description=_GWMH9P5.ELK.000_14_  Inputs=+++
  Add1 : Sum  Description=_GWMH9P5.ELK.000_15_  Inputs=+-
  Add12 : Sum  Description=_GWMH9P5.ELK.000_18_  Inputs=+-
  Add6 : Sum  Description=_GWMH9P5.ELK.000_22_  Inputs=++-
  Add7 : Sum  Description=_GWMH9P5.ELK.000_23_  Inputs=+-
  Add8 : Sum  Description=_GWMH9P5.ELK.000_24_  Inputs=-+
  Constant : Constant  Value=0
  Constant14 : Constant  Description=_GWMH9P4.ELK.000_42_  Value=0
  Constant17 : Constant  Description=_GWMH9P5.ELK.000_26_  Commented=on  OutDataTypeStr=single
  Constant19 : Constant  Description=_GWMH9P5.ELK.000_27_  Commented=on  OutDataTypeStr=single
  Constant2 : Constant  Description=_GWMH9P5.ELK.000_52_  Value=0.85
  Constant3 : Constant  Description=_GWMH9P5.ELK.000_38_  Value=3
  Constant4 : Constant  Description=_GWMH9P5.ELK.000_52_  Value=0.2
  Constant40 : Constant  Description=_GWMH9P5.ELK.000_41_  Commented=on  Value=0.1  mask(TL_Constant){data={'date',738384.85978439811}}
      settings: CopyFcn=if exist('tl_reset_reviewflag','file')==2,tl_reset_reviewflag(gcbh);end  DeleteFcn=if exist('delete_tlblock','file')==2,delete_tlblock(gcbh);end  NameChangeFcn=if exist('rename_tlblock','file')==2,rename_tlblock(gcbh);end  OpenFcn=if exist('tl_open_blockdlg','file')==2,tl_open_blockdlg(gcbh);else,open_system(gcbh,'parameter');end
      mask Help: eval(['if exist(''tl_online_help'',''file'') == 2, tl_online_help(''Topic'',''TL_Constant''),else, web([''jar:file:///'',matlabroot,''/help/toolbox/simulink/help.jar!/slref/' lower(get_param(gcbh,'Blocktype')) '.html''], ''-helpbrowser''),end'])
      mask Display: plot([-0.1, 1.05, NaN, -0.1, 1.05],[.01, .01, NaN, .99, .99]);
  Constant41 : Constant  Description=_GWMH9P5.ELK.000_42_  Commented=on  Value=100  mask(TL_Constant){data={'date',738384.85978776624}}
      settings: CopyFcn=if exist('tl_reset_reviewflag','file')==2,tl_reset_reviewflag(gcbh);end  DeleteFcn=if exist('delete_tlblock','file')==2,delete_tlblock(gcbh);end  NameChangeFcn=if exist('rename_tlblock','file')==2,rename_tlblock(gcbh);end  OpenFcn=if exist('tl_open_blockdlg','file')==2,tl_open_blockdlg(gcbh);else,open_system(gcbh,'parameter');end
      mask Help: eval(['if exist(''tl_online_help'',''file'') == 2, tl_online_help(''Topic'',''TL_Constant''),else, web([''jar:file:///'',matlabroot,''/help/toolbox/simulink/help.jar!/slref/' lower(get_param(gcbh,'Blocktype')) '.html''], ''-helpbrowser''),end'])
      mask Display: plot([-0.1, 1.05, NaN, -0.1, 1.05],[.01, .01, NaN, .99, .99]);
  Constant42 : Constant  Description=_GWMH9P5.ELK.000_43_  Value=0
  Constant43 : Constant  Description=_GWMH9P5.ELK.000_44_  Commented=on  Value=100  mask(TL_Constant){data={'date',738384.85979500005}}
      settings: CopyFcn=if exist('tl_reset_reviewflag','file')==2,tl_reset_reviewflag(gcbh);end  DeleteFcn=if exist('delete_tlblock','file')==2,delete_tlblock(gcbh);end  NameChangeFcn=if exist('rename_tlblock','file')==2,rename_tlblock(gcbh);end  OpenFcn=if exist('tl_open_blockdlg','file')==2,tl_open_blockdlg(gcbh);else,open_system(gcbh,'parameter');end
      mask Help: eval(['if exist(''tl_online_help'',''file'') == 2, tl_online_help(''Topic'',''TL_Constant''),else, web([''jar:file:///'',matlabroot,''/help/toolbox/simulink/help.jar!/slref/' lower(get_param(gcbh,'Blocktype')) '.html''], ''-helpbrowser''),end'])
      mask Display: plot([-0.1, 1.05, NaN, -0.1, 1.05],[.01, .01, NaN, .99, .99]);
  Constant44 : Constant  Description=_GWMH9P5.ELK.000_45_  Commented=on  Value=-0.1  mask(TL_Constant){data={'date',738384.85979850695}}
      settings: CopyFcn=if exist('tl_reset_reviewflag','file')==2,tl_reset_reviewflag(gcbh);end  DeleteFcn=if exist('delete_tlblock','file')==2,delete_tlblock(gcbh);end  NameChangeFcn=if exist('rename_tlblock','file')==2,rename_tlblock(gcbh);end  OpenFcn=if exist('tl_open_blockdlg','file')==2,tl_open_blockdlg(gcbh);else,open_system(gcbh,'parameter');end
      mask Help: eval(['if exist(''tl_online_help'',''file'') == 2, tl_online_help(''Topic'',''TL_Constant''),else, web([''jar:file:///'',matlabroot,''/help/toolbox/simulink/help.jar!/slref/' lower(get_param(gcbh,'Blocktype')) '.html''], ''-helpbrowser''),end'])
      mask Display: plot([-0.1, 1.05, NaN, -0.1, 1.05],[.01, .01, NaN, .99, .99]);
  Constant47 : Constant  Description=_GWMH9P5.ELK.000_48_  Value=5
  Constant49 : Constant  Description=_GWMH9P5.ELK.000_50_  Value=3
  Constant5 : Constant  Description=_GWMH9P5.ELK.000_27_  Commented=on  Value=0.1  OutDataTypeStr=single
  Constant51 : Constant  Description=_GWMH9P5.ELK.000_52_  Value=0.1
  Constant6 : Constant  Description=_GWMH9P5.ELK.000_52_  Value=0.2
  Constant7 : Constant  Description=_GWMH9P5.ELK.000_27_  Commented=on  Value=0.1  OutDataTypeStr=single
  Delay_Trig : Subsystem
  Delay_Trig1 : Subsystem
  First_Order_Lag_Filter : Subsystem  Commented=on
  First_Order_Lag_Filter1 : Subsystem  Commented=on
  Gain1 : Gain  Description=_GWMH9P4.ELK.000_64_  Gain=-1
  Gain17 : Gain  Description=_GWMH9P5.ELK.000_80_  Gain=0.5
  Gain2 : Gain  Description=_GWMH9P4.ELK.000_64_  Gain=0.5
  Gain3 : Gain  Description=_GWMH9P4.ELK.000_64_  Gain=0.5
  Gain4 : Gain  Description=_GWMH9P4.ELK.000_64_  Gain=0.5
  Gain5 : Gain  Description=_GWMH9P4.ELK.000_64_  Gain=-1
  Hysteresis : Stateflow chart  TreatAsAtomicUnit=on
  Hysteresis1 : Stateflow chart  Commented=on  TreatAsAtomicUnit=on
  Hysteresis2 : Stateflow chart  Commented=on  TreatAsAtomicUnit=on
  Logical Operator1 : Logic  Description=_GWMH9P5.ELK.000_86_  AllPortsSameDT=off  OutDataTypeStr=boolean
  Logical Operator4 : Logic  Description=_GWMH9P4.ELK.000_68_  AllPortsSameDT=off  OutDataTypeStr=boolean
  Logical Operator5 : Logic  Description=_GWMH9P4.ELK.000_68_  AllPortsSameDT=off  OutDataTypeStr=boolean
  Logical Operator6 : Logic  Description=_GWMH9P5.ELK.000_88_  Operator=NOT  Inputs=1  AllPortsSameDT=off  OutDataTypeStr=boolean
  Product1 : Product  Description=_GWMH9P4.ELK.000_71_  RndMeth=Zero
  Product11 : Product  Description=_GWMH9P5.ELK.000_91_
  Product13 : Product  Description=_GWMH9P5.ELK.000_93_  RndMeth=Zero
  Product14 : Product  Description=_GWMH9P5.ELK.000_94_  RndMeth=Zero
  Product3 : Product  Description=_GWMH9P4.ELK.000_73_  RndMeth=Zero
  Product9 : Product  Description=_GWMH9P5.ELK.000_102_
  Relational Operator13 : RelationalOperator  Description=_GWMH9P5.ELK.000_106_  Commented=on  OutDataTypeStr=boolean  RndMeth=Nearest  mask(TL_RelationalOperator){data={'date',738384.85993935179}}
      settings: DeleteFcn=if exist('delete_tlblock','file')==2,delete_tlblock(gcbh);end  NameChangeFcn=if exist('rename_tlblock','file')==2,rename_tlblock(gcbh);end  OpenFcn=if exist('tl_open_blockdlg','file')==2,tl_open_blockdlg(gcbh);else,open_system(gcbh,'parameter');end
      mask Help: eval(['if exist(''tl_online_help'',''file'') == 2, tl_online_help(''Topic'',''TL_RelationalOperator''),else, web([''jar:file:///'',matlabroot,''/help/toolbox/simulink/help.jar!/slref/' lower(get_param(gcbh,'Blocktype')) '.html''], ''-helpbrowser''),end'])
      mask Display: plot([-0.1, 1.05, NaN, -0.1, 1.05],[.01, .01, NaN, .99, .99]);
  Relational Operator21 : RelationalOperator  Description=_GWMH9P5.ELK.000_108_  OutDataTypeStr=boolean  RndMeth=Nearest
  Relational Operator25 : RelationalOperator  Description=_GWMH9P5.ELK.000_109_  Commented=on  Operator=<  OutDataTypeStr=boolean  RndMeth=Nearest  mask(TL_RelationalOperator){data={'date',738384.85994590283}}
      settings: DeleteFcn=if exist('delete_tlblock','file')==2,delete_tlblock(gcbh);end  NameChangeFcn=if exist('rename_tlblock','file')==2,rename_tlblock(gcbh);end  OpenFcn=if exist('tl_open_blockdlg','file')==2,tl_open_blockdlg(gcbh);else,open_system(gcbh,'parameter');end
      mask Help: eval(['if exist(''tl_online_help'',''file'') == 2, tl_online_help(''Topic'',''TL_RelationalOperator''),else, web([''jar:file:///'',matlabroot,''/help/toolbox/simulink/help.jar!/slref/' lower(get_param(gcbh,'Blocktype')) '.html''], ''-helpbrowser''),end'])
      mask Display: plot([-0.1, 1.05, NaN, -0.1, 1.05],[.01, .01, NaN, .99, .99]);
  Relational Operator30 : RelationalOperator  Description=_GWMH9P5.ELK.000_112_  Operator=<=  OutDataTypeStr=boolean  RndMeth=Nearest
  Relational Operator5 : RelationalOperator  Description=_GWMH9P3.ELK.000_91_  Operator=<  OutDataTypeStr=boolean  RndMeth=Nearest
  Relational Operator7 : RelationalOperator  Description=_GWMH9P4.ELK.000_81_  Operator=<  OutDataTypeStr=boolean  RndMeth=Nearest
  Relational Operator9 : RelationalOperator  Description=_GWMH9P5.ELK.000_114_  Operator=>  OutDataTypeStr=boolean  RndMeth=Nearest
  Sum1 : Sum  Description=_GWMH9P4.ELK.000_83_  Commented=on  Inputs=+---
  Sum2 : Sum  Description=_GWMH9P4.ELK.000_83_  Inputs=+---
  Switch : Switch  Criteria=u2 > Threshold  Threshold=0.5
  Trigger Hold : Subsystem  Commented=on
  Trigger Hold1 : Subsystem  Commented=on
  TA_Right_Flag1 : Outport
  wires:
  [Lateral_Overlap_Right_Flag] -> Switch/3
  Constant/1 -> Switch/2
  Switch/1 -> [ELKOC_TA_Lateral_OK_Flag_Right]
  Gain5/1 -> Product13/2
  [Host_Veh_Width] -> Gain4/1
  Gain2/1 -> Add/2
  Gain1/1 -> Product1/2
  Obj_Right_LatDist_Trans/1 -> [ObjsSelect_LatDist_Trans]
  Delay_Trig/1(Output) -> Hysteresis/1(Input_1)
  Constant4/1 -> Product11/1
  [Host_Veh_Width] -> Product11/2
  Product11/1 -> [veh_width_buffer_h_Right]
  Product9/1 -> [veh_width_buffer_2]
  Constant6/1 -> Product9/1
  [ObjsSelect_Width] -> Product9/2
  [ObjsSelect_LatDist_Trans] -> [ELK_Oncom_2_Lat_L_filtered]
  [TTC_Calculated] -> Product1/1
  [ObjsSelect_Width] -> Gain3/1
  [right_boundary_lat_rate_filtered] -> Gain1/1
  Constant14/1 -> Relational Operator7/2
  [TTC_Calculated] -> Product3/1
  [ELK_Oncom_2_Lat_Vel_filtered] -> Product3/2
  Gain3/1 -> Sum2/2
  Product3/1 -> Sum2/3
  [veh_width_buffer_2] -> Sum2/4
  Product1/1 -> Add/1
  [Host_Veh_Width] -> Gain2/1
  [veh_width_buffer_h_Right] -> Add/3
  Sum2/1 -> Add1/1
  Add/1 -> Add1/2
  Add1/1 -> Relational Operator13/1, Relational Operator7/1
  Logical Operator1/1 -> [Lateral_Overlap_Right_Flag]
  Hysteresis1/1(Flag) -> Trigger Hold/1(Trigger)
  Relational Operator7/1 -> Logical Operator1/1
  Constant40/1 -> Relational Operator13/2
  Relational Operator13/1 -> Hysteresis1/2(Input_2)
  Constant41/1 -> Trigger Hold/2(hold_time)
  [ObjsSelect_Width] -> Gain17/1
  Gain17/1 -> Add6/2
  [TTC_Calculated] -> Product14/1
  [ELK_Oncom_2_Lat_Vel_filtered] -> Product14/2
  Product14/1 -> Add6/3
  Add6/1 -> Add7/1
  [TTC_Calculated] -> Product13/1
  [right_boundary_lat_rate_filtered] -> Gain5/1
  Add7/1 -> Relational Operator25/1, Relational Operator21/1
  Constant42/1 -> Relational Operator21/2
  [ELK_Oncom_2_Lat_L_filtered] -> Add6/1
  Product13/1 -> Add8/2
  Gain4/1 -> Add8/1
  Add8/1 -> Add7/2
  Constant44/1 -> Relational Operator25/2
  Relational Operator21/1 -> Logical Operator1/2
  Relational Operator25/1 -> Hysteresis2/2(Input_2)
  Hysteresis2/1(Flag) -> Trigger Hold1/1(Trigger)
  Constant43/1 -> Trigger Hold1/2(hold_time)
  [Obj_LatVel_To_RightMkr] -> [ELK_Oncom_2_Lat_Vel_filtered]
  [ELK_Oncom_2_Lat_Vel_filtered] -> Abs/1
  Logical Operator6/1 -> [ELKOC_Tar_R_LatRate_OK_Flag]
  Constant2/1 -> Add12/1, Relational Operator9/2
  Constant47/1 -> Delay_Trig/2(Delay_Cnt)
  Relational Operator30/1 -> Delay_Trig1/1(Input)
  Delay_Trig1/1(Output) -> Hysteresis/2(Input_2)
  Constant49/1 -> Delay_Trig1/2(Delay_Cnt)
  Abs/1 -> Relational Operator30/1, Relational Operator9/1
  Add12/1 -> Relational Operator30/2
  Constant51/1 -> Add12/2
  Relational Operator9/1 -> Delay_Trig/1(Input)
  Hysteresis/1(Flag) -> Logical Operator6/1
  Obj_LatVel_To_RightMkr/1 -> [Obj_LatVel_To_RightMkr]
  right_boundary_lat_rate_filtered/1 -> [right_boundary_lat_rate_filtered]
  Host_Veh_Width/1 -> [Host_Veh_Width]
  TTC_Calculated/1 -> [TTC_Calculated]
  ObjsSelect_Width/1 -> [ObjsSelect_Width]
  [TA_Right_Flag] -> TA_Right_Flag1/1
  [Lateral_Overlap_Right_Flag] -> Logical Operator5/2
  Logical Operator5/1 -> Switch/1
  [ELKOC_Tar_R_LatRate_OK_Flag] -> Logical Operator5/1
  Relational Operator5/1 -> Logical Operator4/1
  Logical Operator4/1 -> [TA_Right_Flag]
  [TTC_Calculated] -> Relational Operator5/1
  [ELKOC_TA_Lateral_OK_Flag_Right] -> Logical Operator4/2
  Constant3/1 -> Relational Operator5/2
  Constant5/1 -> First_Order_Lag_Filter/2(filt_tau_msec)
  Constant19/1 -> First_Order_Lag_Filter/1(filt_loop_time_msec)
  Constant7/1 -> First_Order_Lag_Filter1/2(filt_tau_msec)
  Constant17/1 -> First_Order_Lag_Filter1/1(filt_loop_time_msec)
  [ELK_Oncom_2_Lat_L_filtered] -> Sum2/1

== system TosAeb/ELK_Objsel/Target_Selection/If Action Subsystem/ELK_TargetSelection/Left ==
  note: Unit Delay模块放在循环子系统中时，输出其在循环的上一个迭代的输入值，而不是在上一个仿真时间步的值。
  note: 目标朝左侧车道线运动方向(即向右)为正
  a0_LeftLine : Inport
  a1_LeftLine : Inport  Port=2
  a2_LeftLine : Inport  Port=3
  a3_LeftLine : Inport  Port=4
  ObjsSelect_LongiDist : Inport  Port=5
  ObjsSelect_LatDist : Inport  Port=6
  ObjsSelect_LongiVel_abs : Inport  Port=7
  ObjsSelect_LatVel_abs : Inport  Port=8
  ObjsSelect_Width : Inport  Port=9
  left_line_width : Inport  Port=10
  Abs : Abs
  Abs1 : Abs
  Add1 : Sum  Inputs=+++
  Add{2,5,7..8,10} (5 alike) : Sum  Inputs=+-
  Add3 : Sum
  Add4 : Sum  Inputs=-+
  Constant73 : Constant  Value=0
  Constant{74,78,81..82} (4 alike) : Constant
  Divide{1,3..6} (5 alike) : Product  Inputs=**
  Divide2 : Product  Inputs=*/
  Divide7 : Product  Inputs=*/
  Gain : Gain  Gain=0.5
  Gain1 : Gain  Gain=-1
  Gain2 : Gain  Gain=-1
  Math Function1 : Math  Operator=square  SignedPower=on  SaturateOnIntegerOverflow=on
  Relational Operator14 : RelationalOperator  Operator=>  OutDataTypeStr=boolean  RndMeth=Simplest
  Sqrt2 : Sqrt  SaturateOnIntegerOverflow=on
  Sqrt3 : Sqrt  SaturateOnIntegerOverflow=on
  Subsystem : Subsystem
  Subsystem2 : Subsystem
  Switch2 : Switch  Criteria=u2 > Threshold
  Switch3 : Switch  Criteria=u2 > Threshold
  Switch4 : Switch  Criteria=u2 > Threshold
  Unit Delay1 : UnitDelay  SampleTime=-1  HasFrameUpgradeWarning=on
  Obj_LatVel_To_LeftMkr : Outport
  Obj_Left_dist_mkr : Outport  Port=2
  Obj_Left_LatDist_Trans : Outport  Port=3
  wires:
  [a1_LeftLine] -> Subsystem/2(a1)
  [a3_LeftLine] -> Subsystem2/3(a3)
  Math Function1/1 -> Add3/2
  Constant78/1 -> Add3/1
  [ObjsSelect_LongiVel_abs] -> Divide1/1
  [sin_alpha_LeftLine] -> Divide1/2
  [a0_LeftLine] -> Subsystem/1(a0)
  [a3_LeftLine] -> Subsystem/4(a3)
  [a2_LeftLine] -> Subsystem/3(a2)
  [ObjsSelect_LongiDist] -> Subsystem/5(x)
  Subsystem/1(Out1) -> [dy_LeftLine]
  [a2_LeftLine] -> Subsystem2/2(a2)
  [a1_LeftLine] -> Subsystem2/1(a1)
  [ObjsSelect_LongiDist] -> Subsystem2/4(x)
  Subsystem2/1(Out1) -> [Slope_LeftLine]
  Divide5/1 -> Add2/1
  Relational Operator14/1 -> [Obj_Left_Flag]
  Unit Delay1/1 -> Add10/2
  Add5/1 -> Switch3/1
  Gain/1 -> Add8/2
  [cos_alpha_LeftLine] -> Abs/1
  [ObjsSelect_LongiVel_abs] -> Divide4/1
  [sin_alpha_LeftLine] -> Divide4/2
  Add8/1 -> Relational Operator14/1, [Obj_Left_dist_mkr]
  [Obj_Left_dist_mkr] -> Unit Delay1/1, Add10/1
  Abs1/1 -> Add1/3
  Divide7/1 -> Gain2/1
  Switch4/1 -> [Obj_LatVel_To_LeftMkr]
  Constant73/1 -> Relational Operator14/2
  Divide4/1 -> Add2/2
  [ObjsSelect_Width] -> Gain/1
  Switch3/1 -> Switch4/1
  Add7/1 -> Divide6/1
  Gain2/1 -> Switch4/3
  Add4/1 -> Sqrt3/1
  [cos_alpha_LeftLine] -> Divide3/2
  Add1/1 -> [Obj_Left_LatDist_Trans]
  Sqrt3/1 -> [sin_alpha_LeftLine]
  [left_line_width] -> Add1/2
  Add2/1 -> Switch3/3
  [ObjsSelect_LatVel_abs] -> Divide3/1
  [Slope_LeftLine] -> Switch3/2
  [ObjsSelect_LatVel_abs] -> Divide5/1
  [cos_alpha_LeftLine] -> Divide5/2
  Add10/1 -> Divide7/1
  Constant74/1 -> Switch4/2
  Divide2/1 -> Sqrt2/1, Add4/1
  Constant82/1 -> Add4/2
  Sqrt2/1 -> Switch2/1, Gain1/1
  Divide6/1 -> Add1/1, Add8/1
  [Task_Period] -> Divide7/2
  [Slope_LeftLine] -> Switch2/2, Math Function1/1
  Gain1/1 -> Switch2/3
  Constant81/1 -> Divide2/1
  [ObjsSelect_LatDist] -> Add7/1
  Divide1/1 -> Add5/1
  [dy_LeftLine] -> Add7/2
  Abs/1 -> Divide6/2
  Switch2/1 -> [cos_alpha_LeftLine]
  Divide3/1 -> Add5/2
  Add3/1 -> Divide2/2
  [a0_LeftLine] -> Abs1/1
  a0_LeftLine/1 -> [a0_LeftLine]
  ObjsSelect_LatDist/1 -> [ObjsSelect_LatDist]
  a1_LeftLine/1 -> [a1_LeftLine]
  a3_LeftLine/1 -> [a3_LeftLine]
  a2_LeftLine/1 -> [a2_LeftLine]
  ObjsSelect_LongiDist/1 -> [ObjsSelect_LongiDist]
  ObjsSelect_LongiVel_abs/1 -> [ObjsSelect_LongiVel_abs]
  ObjsSelect_LatVel_abs/1 -> [ObjsSelect_LatVel_abs]
  ObjsSelect_Width/1 -> [ObjsSelect_Width]
  left_line_width/1 -> [left_line_width]
  [Obj_LatVel_To_LeftMkr] -> Obj_LatVel_To_LeftMkr/1
  [Obj_Left_dist_mkr] -> Obj_Left_dist_mkr/1
  [Obj_Left_LatDist_Trans] -> Obj_Left_LatDist_Trans/1

== system TosAeb/ELK_Objsel/Target_Selection/If Action Subsystem/ELK_TargetSelection/Right ==
  note: 目标朝右侧车道线运动方向(即向左)为正
  a0_RightLine : Inport
  a1_RightLine : Inport  Port=2
  a2_RightLine : Inport  Port=3
  a3_RightLine : Inport  Port=4
  ObjsSelect_LongiDist : Inport  Port=5
  ObjsSelect_LatDist : Inport  Port=6
  ObjsSelect_LongiVel_abs : Inport  Port=7
  ObjsSelect_LatVel_abs : Inport  Port=8
  ObjsSelect_Width : Inport  Port=9
  right_line_width : Inport  Port=10
  Abs1 : Abs
  Abs3 : Abs
  Add1 : Sum
  Add{6..7,9..11} (5 alike) : Sum  Inputs=+-
  Add2 : Sum  Inputs=-+
  Add4 : Sum  Inputs=+++
  Constant73 : Constant  Value=0
  Constant{74,78,81..82} (4 alike) : Constant
  Divide : Product  Inputs=*/
  Divide1 : Product  Inputs=*/
  Divide{4..5,7,9..10} (5 alike) : Product  Inputs=**
  Gain2 : Gain  Gain=-1
  Gain3 : Gain  Gain=-1
  Gain5 : Gain  Gain=0.5
  Math Function : Math  Operator=square  SignedPower=on  SaturateOnIntegerOverflow=on
  Relational Operator14 : RelationalOperator  Operator=>  OutDataTypeStr=boolean  RndMeth=Simplest
  Sqrt : Sqrt  SaturateOnIntegerOverflow=on
  Sqrt1 : Sqrt  SaturateOnIntegerOverflow=on
  Subsystem2 : Subsystem
  Subsystem3 : Subsystem
  Switch : Switch  Criteria=u2 > Threshold
  Switch2 : Switch  Criteria=u2 > Threshold
  Switch3 : Switch  Criteria=u2 > Threshold
  Unit Delay1 : UnitDelay  SampleTime=-1  HasFrameUpgradeWarning=on
  Obj_LatVel_To_RightMkr : Outport
  Obj_Right_dist_mkr : Outport  Port=2
  Obj_Right_LatDist_Trans : Outport  Port=3
  wires:
  Subsystem2/1(Out1) -> [dy_RightLine]
  Constant82/1 -> Add2/2
  [a3_RightLine] -> Subsystem2/4(a3)
  Math Function/1 -> Add1/2
  Divide/1 -> Sqrt/1, Add2/1
  Gain3/1 -> Switch/3
  Constant78/1 -> Add1/1
  Sqrt1/1 -> [sin_alpha_RightLine]
  [a1_RightLine] -> Subsystem3/1(a1)
  Add2/1 -> Sqrt1/1
  [a2_RightLine] -> Subsystem3/2(a2)
  [a3_RightLine] -> Subsystem3/3(a3)
  [a0_RightLine] -> Subsystem2/1(a0)
  Switch/1 -> [cos_alpha_RightLine]
  Constant81/1 -> Divide/1
  Sqrt/1 -> Gain3/1, Switch/1
  [a2_RightLine] -> Subsystem2/3(a2)
  [ObjsSelect_LongiDist] -> Subsystem2/5(x)
  Add1/1 -> Divide/2
  [Slope_RightLine] -> Switch/2, Math Function/1
  [ObjsSelect_LongiDist] -> Subsystem3/4(x)
  [a1_RightLine] -> Subsystem2/2(a1)
  Switch3/1 -> [Obj_LatVel_To_RightMkr]
  Subsystem3/1(Out1) -> [Slope_RightLine]
  [ObjsSelect_LatVel_abs] -> Divide5/1
  [Obj_Right_dist_mkr] -> Unit Delay1/1, Add7/1
  [sin_alpha_RightLine] -> Divide9/2
  [right_line_width] -> Add4/2
  [ObjsSelect_Width] -> Gain5/1
  [ObjsSelect_LatVel_abs] -> Divide10/1
  [ObjsSelect_LongiVel_abs] -> Divide4/1
  Divide7/1 -> Add4/1, Add10/1
  [cos_alpha_RightLine] -> Divide5/2
  Divide1/1 -> Gain2/1
  Constant73/1 -> Relational Operator14/2
  Switch2/1 -> Switch3/1
  Add4/1 -> [Obj_Right_LatDist_Trans]
  Abs3/1 -> Add4/3
  Divide5/1 -> Add6/2
  Constant74/1 -> Switch3/2
  [Slope_RightLine] -> Switch2/2
  [Task_Period] -> Divide1/2
  Add10/1 -> Relational Operator14/1, [Obj_Right_dist_mkr]
  [sin_alpha_RightLine] -> Divide4/2
  [a0_RightLine] -> Abs3/1
  Add11/1 -> Switch2/1
  Add9/1 -> Divide7/1
  Divide4/1 -> Add6/1
  Gain2/1 -> Switch3/3
  [cos_alpha_RightLine] -> Divide10/2
  Add6/1 -> Switch2/3
  Abs1/1 -> Divide7/2
  [cos_alpha_RightLine] -> Abs1/1
  Unit Delay1/1 -> Add7/2
  Divide9/1 -> Add11/2
  [dy_RightLine] -> Add9/1
  [ObjsSelect_LatDist] -> Add9/2
  Gain5/1 -> Add10/2
  Divide10/1 -> Add11/1
  [ObjsSelect_LongiVel_abs] -> Divide9/1
  Relational Operator14/1 -> [Obj_Right_Flag]
  Add7/1 -> Divide1/1
  a0_RightLine/1 -> [a0_RightLine]
  ObjsSelect_LatDist/1 -> [ObjsSelect_LatDist]
  a1_RightLine/1 -> [a1_RightLine]
  a3_RightLine/1 -> [a3_RightLine]
  a2_RightLine/1 -> [a2_RightLine]
  ObjsSelect_LongiDist/1 -> [ObjsSelect_LongiDist]
  ObjsSelect_LongiVel_abs/1 -> [ObjsSelect_LongiVel_abs]
  ObjsSelect_LatVel_abs/1 -> [ObjsSelect_LatVel_abs]
  ObjsSelect_Width/1 -> [ObjsSelect_Width]
  right_line_width/1 -> [right_line_width]
  [Obj_LatVel_To_RightMkr] -> Obj_LatVel_To_RightMkr/1
  [Obj_Right_dist_mkr] -> Obj_Right_dist_mkr/1
  [Obj_Right_LatDist_Trans] -> Obj_Right_LatDist_Trans/1
